simulink model "HDVSim_BEV_WPT_Truck"  (R2018b)
settings: Solver=ode3  StartTime=0.0  StopTime=inf  FixedStep=sample_time  MaxStep=0.1  RelTol=1e-3  EnableMultiTasking=on
model workspace (MAT data in the model file):
  Anschaffungskosten = [3707764736; 2; 1; 1; 1; 1]
  Bat : struct (value not decoded)
  DrvTrn = 12
  Eigenschaften = [3707764736; 2; 1; 1; 2; 2]
  Electric_Truck = 1
  Elektro_LKW = 1
  Fahrzyklus = 2
  FuelCell : struct (value not decoded)
  Fueltype = 12
  Gewichte = [3707764736; 2; 1; 1; 3; 3]
  Hybrid_LKW = 1
  Hybrid_Truck = 1
  IZ12 = 3600
  Kraftstoff = 12
  SOC_T_EM_completely_available = 0.5
  SOC_addition = 0.4
  SOC_target = 0.85
  Sailing_without_drag_torque = 1
  MCOS : class17 (value not decoded)
  TCO_Anhaenger = 1
  TCO_Trailer = 1
  T_distance_LPS_down = 250
  T_distance_LPS_up = 250
  Temp1 = [1000 1100 1200 1300 1400 1500 1600 1700 1800 1900 2000 2100 ... (41 elements, 1x41)]
  VSim : struct (value not decoded)
  Vehicle = 'BEV_WPT'
  Vorausschauender_Tempomat = 1
  WPT : struct (value not decoded)
  acquisition_costs = [3707764736; 2; 1; 1; 3; 3]
  addition_for_critical_slope = 0.01
  ambient : struct (value not decoded)
  auxiliary_consumers : struct (value not decoded)
  critical_altitude_difference = 50
  cycle : struct (value not decoded)
  dcycle = 2
  distance_1_PPC = 175
  distance_2_PPC = 200
  distance_PPC_altitude_difference = 10000
  driver : struct (value not decoded)
  em : struct (value not decoded)
  em_M_max = 1563
  engine : struct (value not decoded)
  final_drive : struct (value not decoded)
  ifElectric = 1
  ifOptimized = 1
  look_ahead_PPC = 250
  max_distance = 358138
  name = 'Verbrauchssimulation_Elektro_WPT_LKW'
  propertie = [3707764736; 2; 1; 1; 4; 4]
  prospective_cruise_control = 1
  sample_time = 0.1
  tank : struct (value not decoded)
  tires : struct (value not decoded)
  transmission : struct (value not decoded)
  v_PPC_delta = 2.77777777778
  v_max_electrical_drive_only = 13.8888888889
  vehicle : struct (value not decoded)
  vehicle_F_brake_max = 309890.14
  MCOS : class17 (value not decoded)
  x = [1563 13 1 400 10.0500050682 4.34242437682 3 1 0 978 1339 2 ... (15 elements, 1x15)]
values from neighbouring files (symbols this model uses):
  transmission = Transmission_gearing(15.86, 7, 0, 0, final_drive.ratio)    [Parameterizing.m]
symbols that are objects in the model workspace (value not decoded): ambient, auxiliary_consumers, Bat, cycle, driver, em, final_drive, tires, vehicle, WPT
undefined symbols (not defined in the file or in its folder): Ion, Lithium
libraries: simulink x22, powerlib x4, electricdrivelib x1
(part 2 of 3)

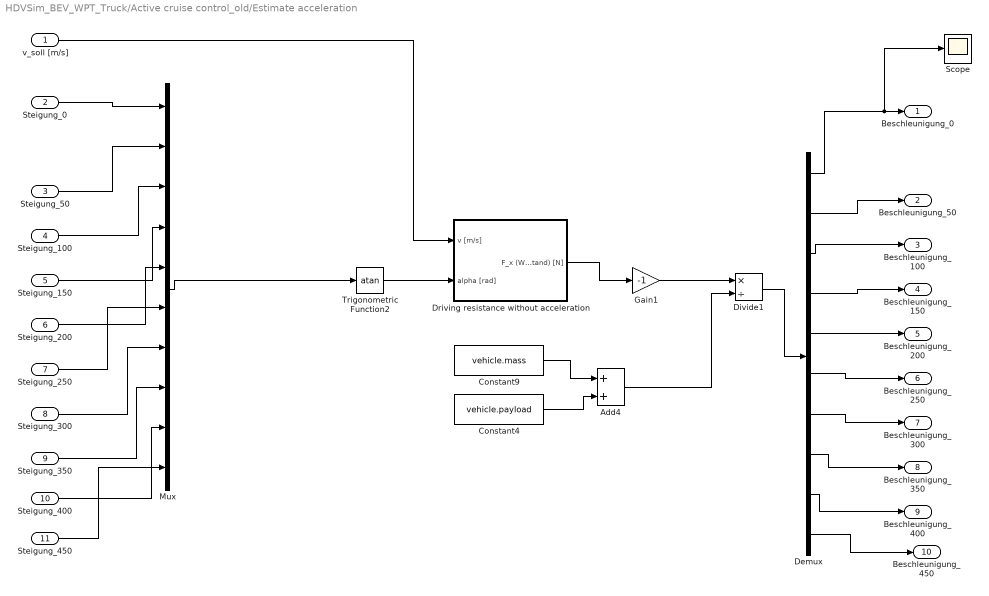
== system HDVSim_BEV_WPT_Truck/Active cruise control_old/Estimate acceleration ==
  v_soll [m/s] : Inport
  Steigung_0 : Inport  Port=2
  Steigung_50 : Inport  Port=3
  Steigung_100 : Inport  Port=4
  Steigung_150 : Inport  Port=5
  Steigung_200 : Inport  Port=6
  "Steigung_250 " : Inport  Port=7
  Steigung_300 : Inport  Port=8
  Steigung_350 : Inport  Port=9
  Steigung_400 : Inport  Port=10
  Steigung_450 : Inport  Port=11
  Add4 : Sum
  Constant4 : Constant  Value=vehicle.payload
  Constant9 : Constant  Value=vehicle.mass
  Demux : Demux  Outputs=10
  Divide1 : Product  Inputs=*/
  Driving resistance without acceleration : Subsystem
  Gain1 : Gain  Gain=-1
  Mux : Mux  Inputs=10
  Scope : Scope
  Trigonometric Function2 : Trigonometry  Operator=atan
  Beschleunigung_0 : Outport
  Beschleunigung_50 : Outport  Port=2
  Beschleunigung_100 : Outport  Port=3
  Beschleunigung_150 : Outport  Port=4
  Beschleunigung_200 : Outport  Port=5
  Beschleunigung_250 : Outport  Port=6
  Beschleunigung_300 : Outport  Port=7
  Beschleunigung_350 : Outport  Port=8
  Beschleunigung_400 : Outport  Port=9
  Beschleunigung_450 : Outport  Port=10
  wires:
  v_soll [m/s]/1 -> Driving resistance without acceleration/1(v [m/s])
  Trigonometric Function2/1 -> Driving resistance without acceleration/2(alpha [rad])
  Constant9/1 -> Add4/1
  Constant4/1 -> Add4/2
  Driving resistance without acceleration/1(F_x (Widerstand) [N]) -> Gain1/1
  Add4/1 -> Divide1/2
  Gain1/1 -> Divide1/1
  Mux/1 -> Trigonometric Function2/1
  Divide1/1 -> Demux/1
  Demux/1 -> Scope/1, Beschleunigung_0/1
  Steigung_0/1 -> Mux/1
  Steigung_50/1 -> Mux/2
  Steigung_100/1 -> Mux/3
  Steigung_150/1 -> Mux/4
  Steigung_200/1 -> Mux/5
  "Steigung_250 "/1 -> Mux/6
  Steigung_300/1 -> Mux/7
  Steigung_350/1 -> Mux/8
  Steigung_400/1 -> Mux/9
  Steigung_450/1 -> Mux/10
  Demux/2 -> Beschleunigung_50/1
  Demux/3 -> Beschleunigung_100/1
  Demux/4 -> Beschleunigung_150/1
  Demux/5 -> Beschleunigung_200/1
  Demux/6 -> Beschleunigung_250/1
  Demux/7 -> Beschleunigung_300/1
  Demux/8 -> Beschleunigung_350/1
  Demux/9 -> Beschleunigung_400/1
  Demux/10 -> Beschleunigung_450/1

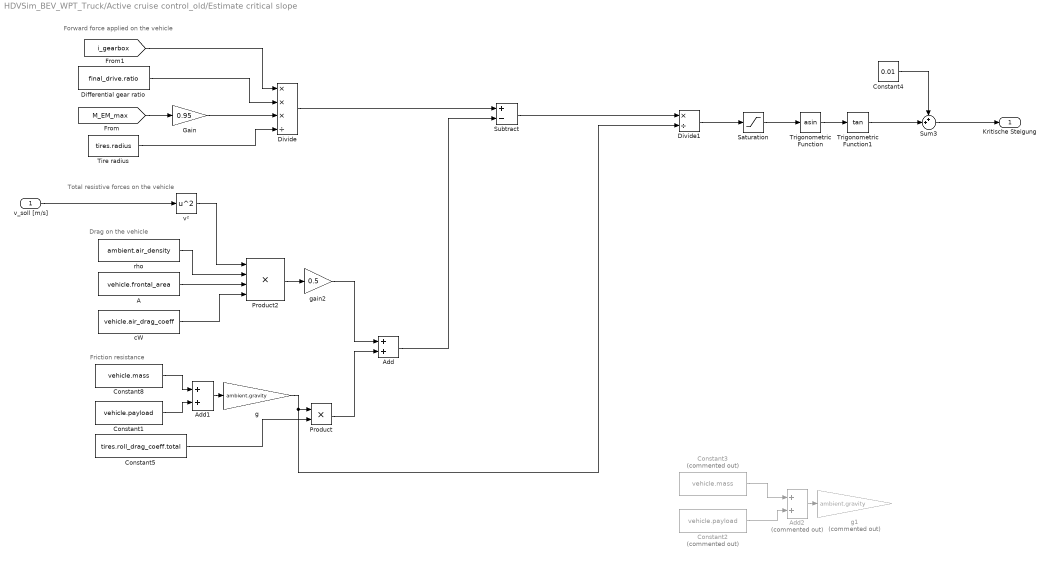
== system HDVSim_BEV_WPT_Truck/Active cruise control_old/Estimate critical slope ==
  note: Forward force applied on the vehicle
  note: Total resistive forces on the vehicle
  note: Drag on the vehicle
  note: Friction resistance
  v_soll [m/s] : Inport
  A : Constant  Value=vehicle.frontal_area
  Add : Sum
  Add1 : Sum
  Add2 : Sum  Commented=on
  Constant1 : Constant  Value=vehicle.payload
  Constant2 : Constant  Commented=on  Value=vehicle.payload
  Constant3 : Constant  Commented=on  Value=vehicle.mass
  Constant4 : Constant  Value=0.01
  Constant5 : Constant  Value=tires.roll_drag_coeff.total
  Constant8 : Constant  Value=vehicle.mass
  Differential gear ratio : Constant  Value=final_drive.ratio
  Divide : Product  Inputs=***/
  Divide1 : Product  Inputs=*/
  Gain : Gain  Gain=0.95
  Product : Product
  Product2 : Product  Inputs=4  RndMeth=Zero
  Saturation : Saturate  UpperLimit=1  LowerLimit=-1
  Subtract : Sum  Inputs=+-
  Sum3 : Sum  Inputs=++|
  Tire radius : Constant  Value=tires.radius
  Trigonometric Function : Trigonometry  Operator=asin
  Trigonometric Function1 : Trigonometry  Operator=tan
  cW : Constant  Value=vehicle.air_drag_coeff
  g : Gain  Gain=ambient.gravity
  g1 : Gain  Commented=on  Gain=ambient.gravity
  gain2 : Gain  Gain=0.5
  rho : Constant  Value=ambient.air_density
  v² : Math  Operator=square  SaturateOnIntegerOverflow=on
  Kritische Steigung : Outport
  wires:
  Gain/1 -> Divide/3
  [i_gearbox] -> Divide/1
  Differential gear ratio/1 -> Divide/2
  Tire radius/1 -> Divide/4
  Divide/1 -> Subtract/1
  v²/1 -> Product2/1
  rho/1 -> Product2/2
  A/1 -> Product2/3
  cW/1 -> Product2/4
  Product2/1 -> gain2/1
  Constant3/1 -> Add2/1
  Constant2/1 -> Add2/2
  Add2/1 -> g1/1
  Trigonometric Function/1 -> Trigonometric Function1/1
  Divide1/1 -> Saturation/1
  Sum3/1 -> Kritische Steigung/1
  [M_EM_max] -> Gain/1
  Constant4/1 -> Sum3/1
  Trigonometric Function1/1 -> Sum3/2
  v_soll [m/s]/1 -> v²/1
  Saturation/1 -> Trigonometric Function/1
  Add1/1 -> g/1
  Constant1/1 -> Add1/2
  g/1 -> Divide1/2, Product/1
  Constant5/1 -> Product/2
  Constant8/1 -> Add1/1
  Product/1 -> Add/2
  gain2/1 -> Add/1
  Add/1 -> Subtract/2
  Subtract/1 -> Divide1/1

== system HDVSim_BEV_WPT_Truck/Driver controls/PI controller brake1 ==
  delta_v : Inport
  Add : Sum
  "I - controller " : Gain  Gain=driver.controller_integral_gain
  "Integrator, reset for sign change of delta v" : Integrator  ExternalReset=either  ZeroCross=off
  "P - controller " : Gain  Gain=driver.controller_proportional_gain
  F_target [N] : Outport
  wires:
  "Integrator, reset for sign change of delta v"/1 -> Add/2  "I_part"
  "I - controller "/1 -> "Integrator, reset for sign change of delta v"/1
  Add/1 -> F_target [N]/1  "F_target"
  "P - controller "/1 -> Add/1  "P_part"
  delta_v/1 -> "I - controller "/1, "Integrator, reset for sign change of delta v"/2, "P - controller "/1

== system HDVSim_BEV_WPT_Truck/Driver controls/PI controller throttle1 ==
  delta_v : Inport
  Add : Sum
  "I - controller " : Gain  Gain=driver.controller_integral_gain
  "Integrator, reset for sign change of delta v" : Integrator  ExternalReset=either  ZeroCross=off
  "P - controller " : Gain  Gain=driver.controller_proportional_gain
  F_target [N] : Outport
  wires:
  "Integrator, reset for sign change of delta v"/1 -> Add/2  "I_part"
  "I - controller "/1 -> "Integrator, reset for sign change of delta v"/1
  Add/1 -> F_target [N]/1  "F_target"
  "P - controller "/1 -> Add/1  "P_partl"
  delta_v/1 -> "Integrator, reset for sign change of delta v"/2, "I - controller "/1, "P - controller "/1

== system HDVSim_BEV_WPT_Truck/Driver controls/ZKU ==
  In1 : Inport
  Switch1 : Switch  Threshold=1
  zero1 : Constant  Value=0
  Out1 : Outport
  wires:
  zero1/1 -> Switch1/1
  [ZKU] -> Switch1/2
  Switch1/1 -> Out1/1
  In1/1 -> Switch1/3

== system HDVSim_BEV_WPT_Truck/Driver controls/ZKU1 ==
  In1 : Inport
  Switch1 : Switch  Threshold=1
  zero1 : Constant  Value=0
  Out1 : Outport
  wires:
  In1/1 -> Switch1/3
  Switch1/1 -> Out1/1
  [ZKU] -> Switch1/2
  zero1/1 -> Switch1/1

== system HDVSim_BEV_WPT_Truck/Driving cycle/Holding time at the beginning ==
  In1 : Inport
  Clock2 : Clock
  Constant3 : Constant  Value=cycle.stop_start
  Constant4 : Constant  Value=0
  Subtract1 : Sum  Inputs=+-
  Switch1 : Switch
  Out1 : Outport
  wires:
  In1/1 -> Switch1/1
  Constant3/1 -> Subtract1/2
  Clock2/1 -> Subtract1/1
  Constant4/1 -> Switch1/3
  Subtract1/1 -> Switch1/2
  Switch1/1 -> Out1/1

== system HDVSim_BEV_WPT_Truck/Driving cycle/Holding time at the end ==
  In1 : Inport
  Clock1 : Clock
  Constant : Constant
  Constant1 : Constant  Value=cycle.stop_end
  Constant2 : Constant
  Hit Crossing : HitCross  HitCrossingDirection=rising
  Integrator : Integrator  ExternalReset=rising
  Subtract : Sum  Inputs=+-
  Sum1 : Sum  Inputs=|++
  Switch : Switch  Criteria=u2 > Threshold  Threshold=cycle.stop_end
  Out1 : Outport
  wires:
  In1/1 -> Integrator/2
  Constant/1 -> Integrator/1
  Subtract/1 -> Hit Crossing/1
  Integrator/1 -> Switch/1
  Hit Crossing/1 -> Out1/1
  Clock1/1 -> Switch/2
  Constant1/1 -> Sum1/2, Subtract/2
  Sum1/1 -> Switch/3
  Switch/1 -> Subtract/1
  Constant2/1 -> Sum1/1

== system HDVSim_BEV_WPT_Truck/Driving cycle/Significant speed deviation ==
  In1 : Inport
  Clock : Clock
  Compare To Constant : simulink/Logic and Bit Operations/Compare To Constant  relop=>=  const=30  OutDataTypeStr=boolean
  Compare To Constant1 : simulink/Logic and Bit Operations/Compare To Constant  relop=>=  const=1000  OutDataTypeStr=boolean
  Compare To Constant2 : simulink/Logic and Bit Operations/Compare To Constant  relop=<=  const=0.1  OutDataTypeStr=boolean
  Compare To Constant3 : simulink/Logic and Bit Operations/Compare To Constant  relop=>=  const=1000  OutDataTypeStr=boolean
  Constant : Constant
  Constant1 : Constant  Value=0
  Constant2 : Constant
  Constant3 : Constant  Value=0
  Display : Display
  Display1 : Display
  Display2 : Display
  Gain : Gain  Gain=3.6
  Gain1 : Gain  Gain=3.6
  Integrator1 : Integrator  ExternalReset=either
  Integrator2 : Integrator  ExternalReset=either
  Logical Operator : Logic  Operator=OR  AllPortsSameDT=off  OutDataTypeStr=boolean
  Sum : Sum  Inputs=|+-
  Switch : Switch  Criteria=u2 > Threshold
  Switch1 : Switch  Criteria=u2 > Threshold
  stop_time : Lookup_n-D  NumberOfTableDimensions=1  BreakpointsForDimension1=cycle.distance  Table=cycle.stop_time  InterpMethod=Flat  RndMeth=Simplest  InputSameDT=on
  Out1 : Outport
  wires:
  In1/1 -> stop_time/1
  stop_time/1 -> Sum/2
  [delta_v] -> Gain/1
  [v_current1] -> Gain1/1
  Gain/1 -> Compare To Constant/1
  Integrator2/1 -> Display/1, Compare To Constant1/1
  Compare To Constant1/1 -> Logical Operator/2
  Gain1/1 -> Compare To Constant2/1
  Integrator1/1 -> Sum/1
  Sum/1 -> Display1/1, Compare To Constant3/1
  Compare To Constant3/1 -> Logical Operator/1
  Logical Operator/1 -> Out1/1
  Switch1/1 -> Integrator2/1, Integrator2/2
  Switch/1 -> Integrator1/2, Integrator1/1
  Constant/1 -> Switch/1
  Compare To Constant2/1 -> Switch/2
  Constant1/1 -> Switch/3
  Constant2/1 -> Switch1/1
  Compare To Constant/1 -> Switch1/2
  Constant3/1 -> Switch1/3
  Clock/1 -> Display2/1

== system HDVSim_BEV_WPT_Truck/ECU/Approach to stop ==
  stop_time [s] : Inport
  Clock : Clock
  Compare To Constant1 : simulink/Logic and Bit Operations/Compare To Constant  relop=<=  const=3  OutDataTypeStr=boolean
  Compare To Zero : simulink/Logic and Bit Operations/Compare To Zero  relop=<=  OutDataTypeStr=boolean
  Constant : Constant
  Constant1 : Constant  Value=3.1
  Constant2 : Constant
  Hit Crossing : HitCross  HitCrossingDirection=rising
  Integrator : Integrator  ExternalReset=rising  InitialCondition=0.001
  Integrator1 : Integrator  ExternalReset=rising
  Scope : Scope  NumInputPorts=2
  Subtract : Sum  Inputs=+-
  Switch : Switch  Criteria=u2 > Threshold  Threshold=3
  Driver_M : Outport
  Driver_B : Outport  Port=2
  wires:
  Constant/1 -> Integrator/1
  stop_time [s]/1 -> Integrator/2, Subtract/2
  Integrator/1 -> Subtract/1
  Subtract/1 -> Compare To Zero/1, Hit Crossing/1
  Constant2/1 -> Integrator1/1
  Hit Crossing/1 -> Integrator1/2
  Clock/1 -> Switch/2
  Constant1/1 -> Switch/3
  Integrator1/1 -> Switch/1
  Switch/1 -> Compare To Constant1/1
  Compare To Constant1/1 -> Scope/1, Driver_M/1
  Compare To Zero/1 -> Scope/2, Driver_B/1

== system HDVSim_BEV_WPT_Truck/ECU/Calculations for braking ==
  M_EM_max [Nm] : Inport
  Driver_B : Inport  Port=2
  Gear : Inport  Port=3
  Differential efficiency : Constant  Value=final_drive.trq_eff
  Differential gear ratio : Constant  Value=final_drive.ratio
  Divide : Product  Inputs=*/
  Divide1 : Product  Inputs=**/*/
  Efficiencies : Lookup  InputValues=[1:length(transmission.ratios)]  Table=transmission.trq_eff  LookUpMeth=Use Input Nearest
  Gearing : Lookup  InputValues=[1:length(transmission.ratios)]  Table=transmission.ratios  LookUpMeth=Use Input Nearest
  Maximum breaking force [N] : Constant  Value=vehicle.F_brake_max
  Product : Product
  Time radius : Constant  Value=tires.radius
  F_brake_required [N] : Outport
  F_brake_elec_max [N] : Outport  Port=2
  wires:
  M_EM_max [Nm]/1 -> Divide1/1
  Divide/1 -> F_brake_elec_max [N]/1
  Divide1/1 -> Divide/1
  Time radius/1 -> Divide/2
  Driver_B/1 -> Product/1
  Maximum breaking force [N]/1 -> Product/2
  Product/1 -> F_brake_required [N]/1
  Gear/1 -> Efficiencies/1, Gearing/1
  Gearing/1 -> Divide1/2
  Efficiencies/1 -> Divide1/3
  Differential gear ratio/1 -> Divide1/4
  Differential efficiency/1 -> Divide1/5

== system HDVSim_BEV_WPT_Truck/ECU/Calculations for throttle ==
  Driver_M : Inport
  SOC : Inport  Port=2
  n_EM [rpm] : Inport  Port=3
  Memory1 : Memory
  Product : Product
  Product1 : Product
  Speed-torque characteristics : Lookup_n-D  NumberOfTableDimensions=1  BreakpointsForDimension1=em.speed  Table=em.trq  ExtrapMethod=Clip  RndMeth=Simplest  InputSameDT=on
  M_EM_max [Nm] : Outport
  M_Required [Nm] : Outport  Port=2
  wires:
  Product1/1 -> Product/2
  Driver_M/1 -> Product/1
  Product/1 -> M_Required [Nm]/1
  Speed-torque characteristics/1 -> Product1/1, M_EM_max [Nm]/1
  SOC/1 -> Product1/2
  n_EM [rpm]/1 -> Memory1/1
  Memory1/1 -> Speed-torque characteristics/1

== system HDVSim_BEV_WPT_Truck/Electric motor/Machine current demand ==
  note: Electric power calculation
  "n_EM " : Inport
  M_EM_target [Nm] : Inport  Port=2
  Eff_EM : Inport  Port=3
  U_Bat [V] : Inport  Port=4
  Divide : Product  Inputs=*/*
  Divide1 : Product  Inputs=*/
  Gain : Gain  Gain=2*pi/60
  Saturation1 : Saturate  UpperLimit=inf  LowerLimit=1
  I_target [A] : Outport
  wires:
  Divide/1 -> Divide1/1
  Divide1/1 -> I_target [A]/1
  Gain/1 -> Divide/3
  M_EM_target [Nm]/1 -> Divide/1
  "n_EM "/1 -> Saturation1/1
  U_Bat [V]/1 -> Divide1/2
  Eff_EM/1 -> Divide/2
  Saturation1/1 -> Gain/1

== system HDVSim_BEV_WPT_Truck/Electric motor/Machine torque demand ==
  n_EM [rpm] : Inport
  I_current [A] : Inport  Port=2
  Eff_EM : Inport  Port=3
  U_Bat [V] : Inport  Port=4
  Divide : Product  Inputs=*/*
  Gain : Gain  Gain=2*pi/60
  Product : Product
  Saturation1 : Saturate  UpperLimit=inf  LowerLimit=1
  M_EM [Nm] : Outport
  wires:
  U_Bat [V]/1 -> Product/1
  n_EM [rpm]/1 -> Saturation1/1
  Product/1 -> Divide/1
  Gain/1 -> Divide/2
  Divide/1 -> M_EM [Nm]/1
  I_current [A]/1 -> Product/2
  Eff_EM/1 -> Divide/3
  Saturation1/1 -> Gain/1

== system HDVSim_BEV_WPT_Truck/Power electronics and battery/Conversion of SOC ==
  SOC_absolut : Inport
  Constant : Constant  Value=0.5-Bat.Useable_range/2
  Constant1 : Constant  Value=0.5+Bat.Useable_range/2
  Divide : Product  Inputs=*/
  Saturation : Saturate  UpperLimit=1  LowerLimit=0
  Sum : Sum  Inputs=+-
  Sum1 : Sum  Inputs=-+
  SOC_relative : Outport
  wires:
  SOC_absolut/1 -> Sum/1
  Constant/1 -> Sum1/1, Sum/2
  Sum/1 -> Divide/1
  Constant1/1 -> Sum1/2
  Sum1/1 -> Divide/2
  Divide/1 -> Saturation/1
  Saturation/1 -> SOC_relative/1

== system HDVSim_BEV_WPT_Truck/Power electronics and battery/Power electronics ==
  I_PE_current [A] : Inport
  1/Eta_LE : Gain  Gain=1/Bat.Efficiency_power_electronics
  Eta_LE : Gain  Gain=Bat.Efficiency_power_electronics
  Switch : Switch  Criteria=u2 > Threshold
  I_BAT_current [A] : Outport
  wires:
  Eta_LE/1 -> Switch/1
  1/Eta_LE/1 -> Switch/3
  I_PE_current [A]/1 -> 1/Eta_LE/1, Eta_LE/1, Switch/2
  Switch/1 -> I_BAT_current [A]/1

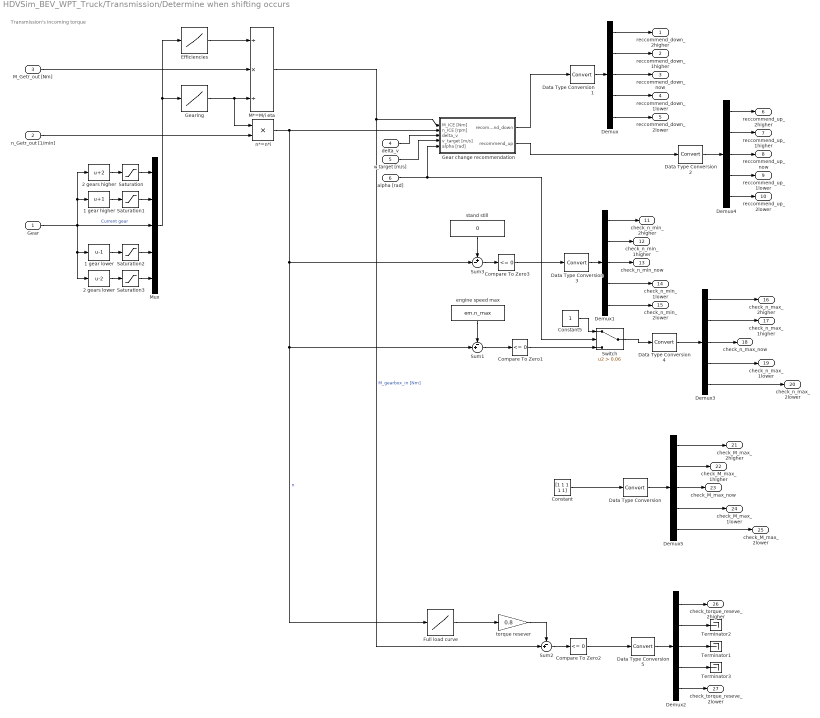
== system HDVSim_BEV_WPT_Truck/Transmission/Determine when shifting occurs ==
  note: Transmission's incoming torque
  Gear : Inport
  n_Getr_out [1/min] : Inport  Port=2
  M_Getr_out [Nm] : Inport  Port=3
  delta_v : Inport  Port=4
  v_target [m/s] : Inport  Port=5
  alpha [rad] : Inport  Port=6
  1 gear higher : Bias  Bias=1
  1 gear lower : Bias  Bias=-1
  2 gears higher : Bias  Bias=2
  2 gears lower : Bias  Bias=-2
  Compare To Zero1 : simulink/Logic and Bit Operations/Compare To Zero  relop=<=  OutDataTypeStr=boolean
  Compare To Zero2 : simulink/Logic and Bit Operations/Compare To Zero  relop=<=  OutDataTypeStr=boolean
  Compare To Zero3 : simulink/Logic and Bit Operations/Compare To Zero  relop=<=  OutDataTypeStr=boolean
  Constant : Constant  Value=[1 1 1 1 1]
  Constant5 : Constant
  Data Type Conversion{,1..5} (6 alike) : DataTypeConversion
  Demux{,1..5} (6 alike) : Demux  Outputs=5
  Efficiencies : Lookup  InputValues=[1:length(transmission.ratios)]  Table=transmission.trq_eff  LookUpMeth=Use Input Nearest
  Full load curve : Lookup  InputValues=em.speed  Table=em.trq  LookUpMeth=Interpolation-Use End Values
  Gear change recommendation : Subsystem
  Gearing : Lookup  InputValues=[1:length(transmission.ratios)]  Table=transmission.ratios  LookUpMeth=Use Input Nearest
  M*=M/i eta : Product  Inputs=/*/
  Mux : Mux  Inputs=5
  Saturation{,1..3} (4 alike) : Saturate  UpperLimit=length(transmission.ratios)  LowerLimit=0
  Sum1 : Sum  Inputs=-+|
  Sum2 : Sum  Inputs=-+|
  Sum3 : Sum  Inputs=+-|
  Switch : Switch  Criteria=u2 > Threshold  Threshold=0.06
  Terminator1 : Terminator
  Terminator2 : Terminator
  Terminator3 : Terminator
  engine speed max : Constant  Value=em.n_max
  n*=n*i : Product  Inputs=**
  stand still : Constant  Value=0
  torque resever : Gain  Gain=0.8
  reccommend_down_2higher : Outport
  reccommend_down_1higher : Outport  Port=2
  reccommend_down_now : Outport  Port=3
  reccommend_down_1lower : Outport  Port=4
  reccommend_down_2lower : Outport  Port=5
  reccommend_up_2higher : Outport  Port=6
  reccommend_up_1higher : Outport  Port=7
  reccommend_up_now : Outport  Port=8
  reccommend_up_1lower : Outport  Port=9
  reccommend_up_2lower : Outport  Port=10
  check_n_min_2higher : Outport  Port=11
  check_n_min_1higher : Outport  Port=12
  check_n_min_now : Outport  Port=13
  check_n_min_1lower : Outport  Port=14
  check_n_min_2lower : Outport  Port=15
  check_n_max_2higher : Outport  Port=16
  check_n_max_1higher : Outport  Port=17
  check_n_max_now : Outport  Port=18
  check_n_max_1lower : Outport  Port=19
  check_n_max_2lower : Outport  Port=20
  check_M_max_2higher : Outport  Port=21
  check_M_max_1higher : Outport  Port=22
  check_M_max_now : Outport  Port=23
  check_M_max_1lower : Outport  Port=24
  check_M_max_2lower : Outport  Port=25
  check_torque_reseve_2higher : Outport  Port=26
  check_torque_reseve_2lower : Outport  Port=27
  wires:
  Gear/1 -> 1 gear higher/1, 2 gears higher/1, Mux/3, 1 gear lower/1, 2 gears lower/1  "Current gear"
  n*=n*i/1 -> Full load curve/1, Sum1/2, Sum3/2, Gear change recommendation/2(n_ICE [rpm])  "n"
  n_Getr_out [1/min]/1 -> n*=n*i/2
  Efficiencies/1 -> M*=M/i eta/1
  M_Getr_out [Nm]/1 -> M*=M/i eta/2
  2 gears higher/1 -> Saturation/1
  1 gear higher/1 -> Saturation1/1
  Mux/1 -> Efficiencies/1, Gearing/1
  Demux/1 -> reccommend_down_2higher/1
  Demux/2 -> reccommend_down_1higher/1
  Demux/3 -> reccommend_down_now/1
  Demux2/1 -> check_torque_reseve_2higher/1
  Demux2/2 -> Terminator2/1
  Demux2/3 -> Terminator1/1
  Demux1/1 -> check_n_min_2higher/1
  Demux1/2 -> check_n_min_1higher/1
  Demux1/3 -> check_n_min_now/1
  Gearing/1 -> n*=n*i/1, M*=M/i eta/3
  Saturation/1 -> Mux/1
  Saturation1/1 -> Mux/2
  1 gear lower/1 -> Saturation2/1
  Saturation2/1 -> Mux/4
  2 gears lower/1 -> Saturation3/1
  Saturation3/1 -> Mux/5
  Demux2/4 -> Terminator3/1
  Demux2/5 -> check_torque_reseve_2lower/1
  Demux/5 -> reccommend_down_2lower/1
  Demux/4 -> reccommend_down_1lower/1
  Demux1/4 -> check_n_min_1lower/1
  Demux1/5 -> check_n_min_2lower/1
  Demux4/1 -> reccommend_up_2higher/1
  Demux4/2 -> reccommend_up_1higher/1
  Demux4/3 -> reccommend_up_now/1
  Demux4/5 -> reccommend_up_2lower/1
  Demux4/4 -> reccommend_up_1lower/1
  Gear change recommendation/1(recommend_down) -> Data Type Conversion1/1
  Full load curve/1 -> torque resever/1
  torque resever/1 -> Sum2/1
  Sum2/1 -> Compare To Zero2/1
  Compare To Zero2/1 -> Data Type Conversion5/1
  Sum3/1 -> Compare To Zero3/1
  stand still/1 -> Sum3/1
  Compare To Zero3/1 -> Data Type Conversion3/1
  Gear change recommendation/2(recommend_up) -> Data Type Conversion2/1
  Demux3/1 -> check_n_max_2higher/1
  Demux3/2 -> check_n_max_1higher/1
  Demux3/3 -> check_n_max_now/1
  Demux3/4 -> check_n_max_1lower/1
  Demux3/5 -> check_n_max_2lower/1
  Sum1/1 -> Compare To Zero1/1
  engine speed max/1 -> Sum1/1
  Demux5/1 -> check_M_max_2higher/1
  Demux5/2 -> check_M_max_1higher/1
  Demux5/3 -> check_M_max_now/1
  Demux5/4 -> check_M_max_1lower/1
  Demux5/5 -> check_M_max_2lower/1
  Data Type Conversion1/1 -> Demux/1
  Data Type Conversion2/1 -> Demux4/1
  Data Type Conversion3/1 -> Demux1/1
  Data Type Conversion4/1 -> Demux3/1
  Data Type Conversion/1 -> Demux5/1
  Data Type Conversion5/1 -> Demux2/1
  Constant/1 -> Data Type Conversion/1
  M*=M/i eta/1 -> Gear change recommendation/1(M_ICE [Nm]), Sum2/2  "M_gearbox_in [Nm]"
  delta_v/1 -> Gear change recommendation/3(delta_v)
  v_target [m/s]/1 -> Gear change recommendation/4(v_target [m/s])
  alpha [rad]/1 -> Gear change recommendation/5(alpha [rad]), Switch/2
  Constant5/1 -> Switch/1
  Switch/1 -> Data Type Conversion4/1
  Compare To Zero1/1 -> Switch/3

== system HDVSim_BEV_WPT_Truck/Transmission/Torque flow through the transmission ==
  note: Outgoing torque from the transmission
  note: Rotational speed before and after the transmission
  M_gearbox_in [Nm] : Inport
  n_gearbox_out [1/min] : Inport  Port=2
  Gear : Inport  Port=3
  Divide : Product  Inputs=/
  Efficiencies : Lookup  InputValues=[1:length(transmission.ratios)]  Table=transmission.trq_eff  LookUpMeth=Use Input Nearest
  Gearing : Lookup  InputValues=[1:length(transmission.ratios)]  Table=transmission.ratios  LookUpMeth=Use Input Nearest
  M_Mot >= 0? : Switch  ZeroCross=off
  Product : Product  Inputs=***  RndMeth=Zero
  Rotating mass : Lookup  InputValues=[1:length(transmission.ratios)]  Table=transmission.lamda  LookUpMeth=Use Input Nearest
  n*=n*i : Product  Inputs=**
  n_gearbox_in [1/min] : Outport
  M_gearbox_out [Nm] : Outport  Port=2
  i_gearbox : Outport  Port=3
  tag scope: Eta_Transmission=global, lamda=global
  wires:
  Gearing/1 -> n*=n*i/1, i_gearbox/1, Product/3  "i_Getriebe"
  n*=n*i/1 -> n_gearbox_in [1/min]/1
  Rotating mass/1 -> [lamda]  "lambda"
  n_gearbox_out [1/min]/1 -> n*=n*i/2
  Gear/1 -> Rotating mass/1, Gearing/1, Efficiencies/1
  M_gearbox_in [Nm]/1 -> M_Mot >= 0?/2, Product/2
  Product/1 -> M_gearbox_out [Nm]/1
  Efficiencies/1 -> Divide/1, M_Mot >= 0?/1  "Eta_Getriebe"
  Divide/1 -> M_Mot >= 0?/3
  M_Mot >= 0?/1 -> [Eta_Transmission], Product/1

== system HDVSim_BEV_WPT_Truck/Transmission/ZKU ==
  Gear : Inport
  Compare : simulink/Logic and Bit Operations/Compare To Zero  relop=>=  OutDataTypeStr=uint8  ZeroCross=off
  Constant : Constant  Value=-1
  Constant1 : Constant  Value=0
  Data Type Conversion : DataTypeConversion
  Derivative : Derivative
  Integrator : Integrator  ExternalReset=either  InitialCondition=transmission.shift_time
  Manual Switch : ManualSwitch
  tag scope: ZKU=global
  wires:
  Constant/1 -> Integrator/1
  Derivative/1 -> Integrator/2
  Integrator/1 -> Compare/1
  Compare/1 -> Data Type Conversion/1
  Data Type Conversion/1 -> [ZKU]
  Constant1/1 -> Manual Switch/2
  Gear/1 -> Manual Switch/1
  Manual Switch/1 -> Derivative/1

== system HDVSim_BEV_WPT_Truck/Vehicle/Driving resistances without acceleration ==
  alpha [rad] : Inport
  v [m/s] : Inport  Port=2
  Add : Sum  Inputs=+++
  Drag : Subsystem
  Gradient resistance : Subsystem
  Rolling resistance : Subsystem
  F_W [N] : Outport
  tag scope: Air_drag=global, Rolling_drag=global
  wires:
  Drag/1(F_drag [N]) -> [Air_drag], Add/2
  Rolling resistance/1(F_Roll [N]) -> Add/1, [Rolling_drag]
  Add/1 -> F_W [N]/1
  v [m/s]/1 -> Drag/1(v [m/s])
  Gradient resistance/1(F_slope [N]) -> Add/3
  alpha [rad]/1 -> Gradient resistance/1(alpha [rad]), Rolling resistance/1(alpha [rad])

== system HDVSim_BEV_WPT_Truck/Active cruise control/Estimate acceleration/Driving resistance without acceleration ==
  v [m/s] : Inport
  alpha [rad] : Inport  Port=2
  Add : Sum  Inputs=+++
  "Drag " : Subsystem
  Gradient resistance : Subsystem
  Rolling resistance : Subsystem
  F_x (resist) [N] : Outport
  wires:
  "Drag "/1(F_drag [N]) -> Add/2
  Rolling resistance/1(F_Roll [N]) -> Add/1
  Add/1 -> F_x (resist) [N]/1  "F_W"
  v [m/s]/1 -> "Drag "/1(v [m/s])
  Gradient resistance/1(F_slope [N]) -> Add/3
  alpha [rad]/1 -> Gradient resistance/1(alpha [rad]), Rolling resistance/1(alpha [rad])

== system HDVSim_BEV_WPT_Truck/Active cruise control_old/Estimate acceleration/Driving resistance without acceleration ==
  v [m/s] : Inport
  alpha [rad] : Inport  Port=2
  Add : Sum  Inputs=+++
  "Drag " : Subsystem
  Gradient resistance : Subsystem
  Rolling resistance : Subsystem
  F_x (Widerstand) [N] : Outport
  wires:
  "Drag "/1(F_Luft [N]) -> Add/2
  Rolling resistance/1(F_Roll [N]) -> Add/1
  Add/1 -> F_x (Widerstand) [N]/1  "F_W"
  v [m/s]/1 -> "Drag "/1(v [m/s])
  Gradient resistance/1(F_Steig [N]) -> Add/3
  alpha [rad]/1 -> Gradient resistance/1(alpha [rad]), Rolling resistance/1(alpha [rad])

== system HDVSim_BEV_WPT_Truck/Transmission/Determine when shifting occurs/Gear change recommendation ==
  note: Gear change commands
  M_ICE [Nm] : Inport
  n_ICE [rpm] : Inport  Port=2
  delta_v : Inport  Port=3
  v_target [m/s] : Inport  Port=4
  alpha [rad] : Inport  Port=5
  Constant1 : Constant  Value=0
  Constant3 : Constant  Value=em.shift_parameter.n2
  Constant5 : Constant  Value=em.shift_parameter.n6
  M_max [Nm] : Constant  Value=em.shift_parameter.M_max
  Switch : Switch  Criteria=u2 > Threshold  Threshold=0.06
  recommend_down_calcbsfc1 : Subsystem
  recommend_up_calc_bsfc1 : Subsystem
  recommend_down : Outport
  recommend_up : Outport  Port=2
  wires:
  M_max [Nm]/1 -> recommend_up_calc_bsfc1/5(M_max [Nm])  "M_max"
  n_ICE [rpm]/1 -> recommend_up_calc_bsfc1/4(n), recommend_down_calcbsfc1/1(n)
  M_ICE [Nm]/1 -> recommend_up_calc_bsfc1/1(M)
  recommend_down_calcbsfc1/1(Out1) -> recommend_down/1
  Constant3/1 -> recommend_up_calc_bsfc1/3(n_2), recommend_down_calcbsfc1/2(n_2)
  Constant5/1 -> recommend_up_calc_bsfc1/2(n_6)
  delta_v/1 -> recommend_down_calcbsfc1/3(delta_v)
  v_target [m/s]/1 -> recommend_up_calc_bsfc1/6(v_target [m/s])
  Constant1/1 -> Switch/1
  recommend_up_calc_bsfc1/1(recommend_up) -> Switch/3
  Switch/1 -> recommend_up/1
  alpha [rad]/1 -> Switch/2

== system HDVSim_BEV_WPT_Truck/Vehicle/Driving resistances without acceleration/Drag ==
  note: F_Drag = 0.5 * V^2 * rho * A * c_d
  v [m/s] : Inport
  A : Constant  Value=vehicle.frontal_area
  Product2 : Product  Inputs=4  RndMeth=Zero
  c_d : Constant  Value=vehicle.air_drag_coeff
  gain2 : Gain  Gain=0.5
  rho : Constant  Value=ambient.air_density
  v² : Math  Operator=square  SaturateOnIntegerOverflow=on
  F_drag [N] : Outport
  wires:
  v²/1 -> Product2/1
  gain2/1 -> F_drag [N]/1
  v [m/s]/1 -> v²/1
  rho/1 -> Product2/2
  A/1 -> Product2/3
  c_d/1 -> Product2/4
  Product2/1 -> gain2/1

== system HDVSim_BEV_WPT_Truck/Vehicle/Driving resistances without acceleration/Gradient resistance ==
  note: F_slope = (m_tot * g) * sin(alpha)
  alpha [rad] : Inport
  Add : Sum
  Cargo weight : Constant  Value=vehicle.payload
  Product7 : Product  RndMeth=Zero
  Trigonometric Function : Trigonometry
  Vehicle weight : Constant  Value=vehicle.mass
  g : Gain  Gain=ambient.gravity
  F_slope [N] : Outport
  wires:
  alpha [rad]/1 -> Trigonometric Function/1
  Trigonometric Function/1 -> Product7/2
  Product7/1 -> F_slope [N]/1
  g/1 -> Product7/1
  Vehicle weight/1 -> Add/1
  Cargo weight/1 -> Add/2
  Add/1 -> g/1

== system HDVSim_BEV_WPT_Truck/Vehicle/Driving resistances without acceleration/Rolling resistance ==
  note: F_Roll = (m_tot * g) * mu * cos(alpha)
  alpha [rad] : Inport
  Add1 : Sum
  Cargo weight : Constant  Value=vehicle.payload
  Product7 : Product  Inputs=3  RndMeth=Zero
  Rolling friction coefficient : Constant  Value=tires.roll_drag_coeff.total
  Trigonometric Function : Trigonometry  Operator=cos
  Vehicle weight : Constant  Value=vehicle.mass
  g : Gain  Gain=ambient.gravity
  F_Roll [N] : Outport
  wires:
  alpha [rad]/1 -> Trigonometric Function/1
  Product7/1 -> F_Roll [N]/1
  Rolling friction coefficient/1 -> Product7/3
  Trigonometric Function/1 -> Product7/1
  g/1 -> Product7/2
  Vehicle weight/1 -> Add1/1
  Cargo weight/1 -> Add1/2
  Add1/1 -> g/1

== system HDVSim_BEV_WPT_Truck/Active cruise control/Estimate acceleration/Driving resistance without acceleration/Drag  ==
  note: F_Drag = 0.5 * V^2 * rho * A * c_d
  v [m/s] : Inport
  A : Constant  Value=vehicle.frontal_area
  Product2 : Product  Inputs=4  RndMeth=Zero
  c_d : Constant  Value=vehicle.air_drag_coeff(end)
  gain2 : Gain  Gain=0.5
  rho : Constant  Value=ambient.air_density
  v² : Math  Operator=square  SaturateOnIntegerOverflow=on
  F_drag [N] : Outport
  wires:
  v²/1 -> Product2/1
  gain2/1 -> F_drag [N]/1
  v [m/s]/1 -> v²/1
  rho/1 -> Product2/2
  A/1 -> Product2/3
  Product2/1 -> gain2/1
  c_d/1 -> Product2/4

== system HDVSim_BEV_WPT_Truck/Active cruise control/Estimate acceleration/Driving resistance without acceleration/Gradient resistance ==
  note: F_slope = (m_tot * g) * sin(alpha)
  alpha [rad] : Inport
  Add : Sum
  Constant1 : Constant  Value=vehicle.payload
  Constant2 : Constant  Value=vehicle.mass
  Product7 : Product  RndMeth=Zero
  Trigonometric Function : Trigonometry
  g : Gain  Gain=ambient.gravity
  F_slope [N] : Outport
  wires:
  alpha [rad]/1 -> Trigonometric Function/1
  Trigonometric Function/1 -> Product7/2
  Product7/1 -> F_slope [N]/1
  g/1 -> Product7/1
  Constant2/1 -> Add/1
  Constant1/1 -> Add/2
  Add/1 -> g/1

== system HDVSim_BEV_WPT_Truck/Active cruise control/Estimate acceleration/Driving resistance without acceleration/Rolling resistance ==
  note: F_Roll = (m_tot * g) * mu * cos(alpha)
  alpha [rad] : Inport
  Add1 : Sum
  Constant1 : Constant  Value=vehicle.payload
  Constant5 : Constant  Value=tires.roll_drag_coeff.total
  Constant8 : Constant  Value=vehicle.mass
  Product7 : Product  Inputs=3  RndMeth=Zero
  Trigonometric Function : Trigonometry  Operator=cos
  g : Gain  Gain=ambient.gravity
  F_Roll [N] : Outport
  wires:
  alpha [rad]/1 -> Trigonometric Function/1
  Product7/1 -> F_Roll [N]/1
  Constant5/1 -> Product7/3
  Trigonometric Function/1 -> Product7/1
  g/1 -> Product7/2
  Constant8/1 -> Add1/1
  Constant1/1 -> Add1/2
  Add1/1 -> g/1

== system HDVSim_BEV_WPT_Truck/Active cruise control_old/Estimate acceleration/Driving resistance without acceleration/Drag  ==
  note: F_Drag = 0.5 * V^2 * rho * A * c_d
  v [m/s] : Inport
  A : Constant  Value=vehicle.frontal_area
  Product2 : Product  Inputs=4  RndMeth=Zero
  cW : Constant  Value=vehicle.air_drag_coeff
  gain2 : Gain  Gain=0.5
  rho : Constant  Value=ambient.air_density
  v² : Math  Operator=square  SaturateOnIntegerOverflow=on
  F_Luft [N] : Outport
  wires:
  v²/1 -> Product2/1
  gain2/1 -> F_Luft [N]/1
  v [m/s]/1 -> v²/1
  rho/1 -> Product2/2
  A/1 -> Product2/3
  cW/1 -> Product2/4
  Product2/1 -> gain2/1

== system HDVSim_BEV_WPT_Truck/Active cruise control_old/Estimate acceleration/Driving resistance without acceleration/Gradient resistance ==
  note: F_slope = (m_tot * g) * sin(alpha)
  alpha [rad] : Inport
  Add : Sum
  Constant1 : Constant  Value=vehicle.payload
  Constant2 : Constant  Value=vehicle.mass
  Product7 : Product  RndMeth=Zero
  Trigonometric Function : Trigonometry
  g : Gain  Gain=ambient.gravity
  F_Steig [N] : Outport
  wires:
  alpha [rad]/1 -> Trigonometric Function/1
  Trigonometric Function/1 -> Product7/2
  Product7/1 -> F_Steig [N]/1
  g/1 -> Product7/1
  Constant2/1 -> Add/1
  Constant1/1 -> Add/2
  Add/1 -> g/1

== system HDVSim_BEV_WPT_Truck/Active cruise control_old/Estimate acceleration/Driving resistance without acceleration/Rolling resistance ==
  note: F_Roll = (m_tot * g) * mu * cos(alpha)
  alpha [rad] : Inport
  Add1 : Sum
  Constant1 : Constant  Value=vehicle.payload
  Constant5 : Constant  Value=tires.roll_drag_coeff.total
  Constant8 : Constant  Value=vehicle.mass
  Product7 : Product  Inputs=3  RndMeth=Zero
  Trigonometric Function : Trigonometry  Operator=cos
  g : Gain  Gain=ambient.gravity
  F_Roll [N] : Outport
  wires:
  alpha [rad]/1 -> Trigonometric Function/1
  Product7/1 -> F_Roll [N]/1
  Constant5/1 -> Product7/3
  Trigonometric Function/1 -> Product7/1
  g/1 -> Product7/2
  Constant8/1 -> Add1/1
  Constant1/1 -> Add1/2
  Add1/1 -> g/1

== system HDVSim_BEV_WPT_Truck/Transmission/Determine when shifting occurs/Gear change recommendation/recommend_down_calcbsfc1 ==
  n : Inport
  n_2 : Inport  Port=2
  delta_v : Inport  Port=3
  Compare To Constant : simulink/Logic and Bit Operations/Compare To Constant  relop=>=  const=8  OutDataTypeStr=boolean
  Compare To Constant1 : simulink/Logic and Bit Operations/Compare To Constant  relop=>=  const=5  OutDataTypeStr=boolean
  Compare To Zero3 : simulink/Logic and Bit Operations/Compare To Zero  relop=<=  OutDataTypeStr=boolean
  Constant : Constant  Value=em.n_eck
  Constant2 : Constant
  Constant3 : Constant  Value=0
  Display : Display
  Gain : Gain  Gain=3.6
  Gain1 : Gain  Gain=1.05
  Integrator2 : Integrator  ExternalReset=either
  Logical Operator : Logic  Operator=OR  AllPortsSameDT=off  OutDataTypeStr=boolean
  Logical Operator1 : Logic  AllPortsSameDT=off  OutDataTypeStr=boolean
  Relational Operator : RelationalOperator  Operator=<=  OutDataTypeStr=boolean  RndMeth=Nearest
  Scope : Scope  NumInputPorts=3
  Speed jump when shifting down (gear dependent) : Gain  Gain=transmission.ratios(1)/transmission.ratios(2)
  Sum1 : Sum  Inputs=+-|
  Switch1 : Switch  Criteria=u2 > Threshold
  Out1 : Outport
  wires:
  Sum1/1 -> Compare To Zero3/1
  n/1 -> Speed jump when shifting down (gear dependent)/1, Sum1/1
  n_2/1 -> Sum1/2
  Logical Operator/1 -> Scope/3, Out1/1
  Compare To Zero3/1 -> Logical Operator/1
  delta_v/1 -> Gain/1
  Gain/1 -> Compare To Constant/1
  Integrator2/1 -> Display/1, Compare To Constant1/1
  Switch1/1 -> Integrator2/1, Integrator2/2
  Constant2/1 -> Switch1/1
  Compare To Constant/1 -> Switch1/2
  Constant3/1 -> Switch1/3
  Compare To Constant1/1 -> Scope/2, Logical Operator1/1  "velocity too low"
  Logical Operator1/1 -> Logical Operator/2
  Speed jump when shifting down (gear dependent)/1 -> Relational Operator/1
  Constant/1 -> Gain1/1
  Gain1/1 -> Relational Operator/2
  Relational Operator/1 -> Scope/1, Logical Operator1/2  "Speed jump when shifting"

== system HDVSim_BEV_WPT_Truck/Transmission/Determine when shifting occurs/Gear change recommendation/recommend_up_calc_bsfc1 ==
  M : Inport
  n_6 : Inport  Port=2
  n_2 : Inport  Port=3
  n : Inport  Port=4
  M_max [Nm] : Inport  Port=5
  v_target [m/s] : Inport  Port=6
  Compare To Zero : simulink/Logic and Bit Operations/Compare To Zero  relop=<=  OutDataTypeStr=boolean
  Compare To Zero1 : simulink/Logic and Bit Operations/Compare To Zero  relop=~=  OutDataTypeStr=boolean
  Logical Operator : Logic  AllPortsSameDT=off  OutDataTypeStr=boolean
  MATLAB Function1 : MATLAB Function  TreatAsAtomicUnit=on
  Sum2 : Sum  Inputs=+-|
  recommend_up : Outport
  wires:
  n_6/1 -> MATLAB Function1/3(n_6)
  n/1 -> MATLAB Function1/1(n)
  n_2/1 -> MATLAB Function1/4(n_2)
  M_max [Nm]/1 -> MATLAB Function1/2(M_max)
  M/1 -> Sum2/1
  Sum2/1 -> Compare To Zero/1
  MATLAB Function1/1(g_2) -> Sum2/2
  v_target [m/s]/1 -> Compare To Zero1/1
  Compare To Zero/1 -> Logical Operator/1
  Compare To Zero1/1 -> Logical Operator/2
  Logical Operator/1 -> recommend_up/1

== stateflow chart HDVSim_BEV_WPT_Truck/ECU/Chart ==
  state Hybrid_Truck
    state Accelerating
      state Electric_driving
        during: u_EM = M_required / M_EM_max; u_KB = 0;
      (default) -> (junction 496)
      (junction 496) -> Electric_driving
    state Braking
      state Braking1
        % Conventional braking
        during: u_KB = Driver_B; u_EM = 0;
      state Braking2
        % Regenerative braking, additionally possibly conventional braking
        entry: REKUP = 1;
        exit: REKUP = 0;
        state Braking3
          % Regenerative and conventional braking
          during: u_EM = -1; u_KB = (F_brake_required - F_brake_el_max) / vehicle_F_brake_max;
        state Braking4
          % Regenerative braking
          during: u_EM = -(F_brake_required / F_brake_el_max); u_KB = 0;
        (junction 504) -> Braking3 [order 1] : [F_brake_required > F_brake_el_max]
        Braking4 -> Braking3 : [F_brake_required > F_brake_el_max]
        Braking3 -> Braking4 : [F_brake_required <= F_brake_el_max]
        (default) -> (junction 504)
        (junction 504) -> Braking4 [order 2] : [F_brake_required <= F_brake_el_max]
      (junction 497) -> Braking1 [order 1] : [SOC == 1]
      Braking2 -> Braking1 : [SOC == 1]
      Braking1 -> Braking2 : [SOC < 1]
      (default) -> (junction 497)
      (junction 497) -> Braking2 [order 2]
    state Rolling
      entry: ROLL = 1;
      exit: ROLL = 0;
      state Rolling1
        during: u_EM = 0; u_KB = 0;
      (default) -> Rolling1
    (junction 513) -> Accelerating [order 1] : [Driver_M > 0 && Driver_B == 0]
    (default) -> (junction 513)
    (junction 513) -> Braking [order 2] : [Driver_B > 0 && Driver_M == 0]
    (junction 513) -> Rolling [order 3]
    Braking -> Accelerating [order 1] : [Driver_M > 0 && Driver_B == 0]
    Accelerating -> Braking [order 1] : [Driver_B > 0 && Driver_M == 0]
    Rolling -> Accelerating [order 2] : [Driver_M > 0 && Driver_B == 0]
    Accelerating -> Rolling [order 2] : [Driver_M == 0 && Driver_B == 0]
    Rolling -> Braking [order 1] : [Driver_B > 0 && Driver_M == 0]
    Braking -> Rolling [order 2] : [Driver_M == 0 && Driver_B == 0]
  (junction 222) -> Hybrid_Truck : [Hybrid_Truck == 1]
  (default) -> (junction 222)
  data: u_EM output; Driver_M input; Driver_B input; SOC input; M_EM_max input; M_required input; F_brake_required input; F_brake_el_max input; u_KB output; Hybrid_Truck parameter; ROLL output; REKUP output; vehicle_F_brake_max local =vehicle.F_brake_max; em_M_max local double =em.M_max

== stateflow chart HDVSim_BEV_WPT_Truck/Active cruise control_old/Chart ==
  state Steigung
    entry: Signal_Steigung = 1;
    during: v_vT = 6/3.6;
    exit: Signal_Steigung = 0;
  state Ebene
    during: Signal_Steigung = 0; v_vT = 0;
  state Gefaelle1
    entry: Signal_Gefaelle = 1;
    exit: Signal_Gefaelle = 0;
  Ebene -> Steigung [order 2] : [Steigung_500 > Kritische_Steigung &&...
      | Steigung_700 > Kritische_Steigung &&...
      | Vorausschauender_Tempomat == 1]
  Steigung -> Ebene : [Steigung_50 <= Kritische_Steigung &&...
      | Steigung_0 <= Kritische_Steigung &&...
      | Zurueckgelegte_Strecke > 500]
  (default) -> Ebene
  Ebene -> Gefaelle1 [order 1] : [(Beschleunigung_0 +...
      | Beschleunigung_50 +...
      | Beschleunigung_100 +...
      | Beschleunigung_150 +...
      | Beschleunigung_200)/5 >= -0.17 &&...
      | (Beschleunigung_250 +...
      | Beschleunigung_300 +...
      | Beschleunigung_350 +...
      | Beschleunigung_400 +...
      | Beschleunigung_450)/5 >= 0.17 &&...
      | Fahrer_M > 0 &&...
      | Vorausschauender_Tempomat == 1]
  Gefaelle1 -> Ebene : [Zurueckgelegte_Strecke_2 > 450 ||...
      | Fahrer_B > 0]
  data: Kritische_Steigung input; Beschleunigung_0 input; Beschleunigung_50 input; Beschleunigung_100 input; Beschleunigung_150 input; Beschleunigung_200 input; Beschleunigung_250 input; Beschleunigung_300 input; Beschleunigung_350 input; Beschleunigung_400 input; Beschleunigung_450 input; Steigung_0 input; Steigung_50 input; Steigung_100 input; Steigung_250 input; Steigung_350 input; Steigung_500 input; Steigung_700 input; Fahrer_M input; Fahrer_B input; Zurueckgelegte_Strecke input; Zurueckgelegte_Strecke_2 input; v_vT output double; Signal_Steigung output double; Signal_Gefaelle output double; Vorausschauender_Tempomat parameter

== stateflow chart HDVSim_BEV_WPT_Truck/Transmission/Shifting rules ==
  state gear_state [parallel]
    settings: executionOrder=1
    state gear016
      entry: gear=16;
    state gear015
      entry: gear=15;
    state gear014
      entry: gear=14;
    state gear013
      entry: gear=13;
    state gear012
      entry: gear=12;
    state gear011
      entry: gear=11;
    state gear010
      entry: gear=10;
    state gear09
      entry: gear=9;
    state gear08
      entry: gear=8;
    state gear07
      entry: gear=7;
    state gear06
      entry: gear=6;
    state gear05
      entry: gear=5;
    state gear04
      entry: gear=4;
    state gear03
      entry: gear=3;
    state gear02
      entry: gear=2;
    state gear01
      entry: gear=1;
    gear015 -> gear016 [order 3] : TWO_UP
    gear016 -> gear014 [order 2] : TWO_DOWN
    gear014 -> gear016 [order 3] : TWO_UP
    (junction 349) -> gear016 [order 16] : [init_gear==16]
    gear016 -> gear015 [order 1] : DOWN
    gear015 -> gear016 [order 1] : UP
    gear015 -> gear013 [order 4] : TWO_DOWN
    (junction 349) -> gear015 [order 15] : [init_gear==15]
    gear013 -> gear015 [order 3] : TWO_UP
    gear015 -> gear014 [order 2] : DOWN
    gear014 -> gear015 [order 1] : UP
    gear014 -> gear012 [order 4] : TWO_DOWN
    gear012 -> gear014 [order 4] : TWO_UP
    (junction 349) -> gear014 [order 14] : [init_gear==14]
    gear014 -> gear013 [order 2] : DOWN
    gear013 -> gear014 [order 1] : UP
    gear011 -> gear013 [order 4] : TWO_UP
    gear013 -> gear011 [order 4] : TWO_DOWN
    (junction 349) -> gear013 [order 13] : [init_gear==13]
    gear013 -> gear012 [order 2] : DOWN
    gear012 -> gear013 [order 3] : UP
    (junction 349) -> gear012 [order 12] : [init_gear==12]
    gear010 -> gear012 [order 4] : TWO_UP
    gear012 -> gear010 [order 2] : TWO_DOWN
    gear012 -> gear011 [order 1] : DOWN
    gear011 -> gear012 [order 1] : UP
    gear011 -> gear09 [order 3] : TWO_DOWN
    gear09 -> gear011 [order 4] : TWO_UP
    (junction 349) -> gear011 [order 11] : [init_gear==11]
    gear011 -> gear010 [order 2] : DOWN
    gear010 -> gear011 [order 1] : UP
    gear08 -> gear010 [order 4] : TWO_UP
    gear010 -> gear08 [order 3] : TWO_DOWN
    (junction 349) -> gear010 [order 10] : [init_gear==10]
    gear010 -> gear09 [order 2] : DOWN
    gear09 -> gear010 [order 1] : UP
    gear07 -> gear09 [order 4] : TWO_UP
    gear09 -> gear07 [order 3] : TWO_DOWN
    (junction 349) -> gear09 [order 9] : [init_gear==9]
    gear09 -> gear08 [order 2] : DOWN
    gear08 -> gear09 [order 2] : UP
    gear06 -> gear08 [order 4] : TWO_UP
    gear08 -> gear06 [order 3] : TWO_DOWN
    (junction 349) -> gear08 [order 8] : [init_gear==8]
    gear08 -> gear07 [order 1] : DOWN
    gear07 -> gear08 [order 3] : UP
    gear07 -> gear05 [order 2] : TWO_DOWN
    gear05 -> gear07 [order 4] : TWO_UP
    (junction 349) -> gear07 [order 7] : [init_gear==7]
    gear07 -> gear06 [order 1] : DOWN
    gear06 -> gear07 [order 3] : UP
    (junction 349) -> gear06 [order 6] : [init_gear==6]
    gear06 -> gear04 [order 2] : TWO_DOWN
    (junction 349) -> gear01 [order 1] : [init_gear==1]
    (junction 349) -> gear05 [order 5] : [init_gear==5]
    gear04 -> gear06 [order 3] : TWO_UP
    (junction 349) -> gear02 [order 2] : [init_gear==2]
    (junction 349) -> gear04 [order 4] : [init_gear==4]
    (junction 349) -> gear03 [order 3] : [init_gear==3]
    gear06 -> gear05 [order 1] : DOWN
    gear05 -> gear06 [order 1] : UP
    gear05 -> gear03 [order 3] : TWO_DOWN
    gear03 -> gear05 [order 3] : TWO_UP
    gear05 -> gear04 [order 2] : DOWN
    gear04 -> gear05 [order 1] : UP
    gear04 -> gear02 [order 4] : TWO_DOWN
    gear02 -> gear04 [order 3] : TWO_UP
    gear04 -> gear03 [order 2] : DOWN
    gear03 -> gear04 [order 1] : UP
    gear01 -> gear03 [order 2] : TWO_UP
    gear03 -> gear01 [order 4] : TWO_DOWN
    gear03 -> gear02 [order 2] : DOWN
    gear02 -> gear03 [order 1] : UP
    gear02 -> gear01 [order 4] : TWO_DOWN
    gear02 -> gear01 [order 2] : DOWN
    gear01 -> gear02 [order 1] : UP
  state selection_state [parallel]
    settings: executionOrder=2
    state upshift2
      entry: TWO_UP;
      exit: wait_state.WAIT;
    state upshift1
      entry: UP;
      exit: wait_state.WAIT;
    note: 2: UP due to recommendation
        | 3: UP due to speed max
    state steady_state
    note: 1: DOWN due to recommendation
        | 4: DOWN due to torque maximum
        | 5: DOWN due to speed min
    state downshift1
      entry: DOWN;
      exit: wait_state.WAIT;
    state downshift2
      entry: TWO_DOWN;
      exit: wait_state.WAIT;
    upshift2 -> steady_state
    (junction 405) -> upshift2 [order 1] : [recommend_down_2higher==0]
    (junction 405) -> upshift1 [order 2] : [recommend_down_2higher==1]
    (junction 404) -> (junction 405) [order 1] : [check_torque_reserve_2higher==1]
    (junction 404) -> upshift1 [order 2] : [check_torque_reserve_2higher==0]
    (junction 403) -> (junction 404) [order 1] : [check_n_min_2higher==1 && check_M_max_2higher==1]
    (junction 403) -> upshift1 [order 2] : [check_n_min_2higher==0 || check_M_max_2higher==0]
    (junction 380) -> (junction 403) [order 1] : [recommend_up_1higher==1 && gear<=z-2]
    (junction 380) -> upshift1 [order 2] : [recommend_up_1higher==0 || gear>z-2]
    steady_state -> upshift1 [order 3] : [check_n_max_now==0 && gear<=z-1]
    upshift1 -> steady_state
    steady_state -> (junction 380) [order 2] : [(recommend_up_now==1 && check_n_max_now==1) && check_n_min_1higher==1 && check_M_max_1higher==1 && gear<=z-1 && waiting3s==0]
    (default) -> steady_state
    downshift1 -> steady_state
    downshift2 -> steady_state
    steady_state -> (junction 367) [order 1] : [(recommend_down_now==1 && check_M_max_now==1 && check_n_min_now==1) && check_n_max_1lower==1 && gear>=2 && waiting3s==0]
    steady_state -> downshift1 [order 4] : [(check_M_max_now==0 && check_n_min_now==1) && check_n_max_1lower==1 &&gear>=2 && check_n_min_now==1 && waiting3s==0]
    steady_state -> downshift1 [order 5] : [(check_n_min_now==0)&& gear>3]
    (junction 367) -> downshift1 [order 1] : [recommend_down_1lower==0 || gear<3]
    (junction 367) -> (junction 394) [order 2] : [recommend_down_1lower==1 && gear>=3]
    (junction 394) -> downshift1 [order 2] : [check_n_max_2lower==0]
    (junction 394) -> (junction 397) [order 1] : [check_n_max_2lower==1]
    (junction 397) -> downshift1 [order 2] : [recommend_up_2lower==1]
    (junction 397) -> downshift2 [order 1] : [recommend_up_2lower==0]
  state wait_state [parallel]
    settings: executionOrder=3
    state shifting_ready
      entry: waiting3s=0;
    state wait3s
      entry: waiting3s=1;
    shifting_ready -> wait3s : WAIT
    (default) -> shifting_ready
    wait3s -> shifting_ready : after(3,sec)
  (default) -> (junction 349)
  data: gear output uint8; waiting3s local double; init_gear input double; z input double; recommend_down_2higher input boolean; recommend_down_1higher input boolean; recommend_down_now input boolean; recommend_down_1lower input boolean; recommend_down_2llower input boolean; recommend_up_2higher input boolean; recommend_up_1higher input boolean; recommend_up_now input boolean; recommend_up_1lower input boolean; recommend_up_2lower input boolean; check_n_min_2higher input boolean; check_n_min_1higher input boolean; check_n_min_now input boolean; check_n_min_1lower input boolean; check_n_min_2lower input boolean; check_n_max_2higher input boolean; check_n_max_1higher input boolean; check_n_max_now input boolean; check_n_max_1lower input boolean; check_n_max_2lower input boolean; check_M_max_2higher input boolean; check_M_max_1higher input boolean; check_M_max_now input boolean; check_M_max_1lower input boolean; check_M_max_2lower input boolean; check_torque_reserve_2higher input boolean; check_torque_reserve_2lower input boolean
  events: WAIT local EITHER_EDGE_EVENT; DOWN local EITHER_EDGE_EVENT; TWO_DOWN local EITHER_EDGE_EVENT; TWO_UP local EITHER_EDGE_EVENT; UP local EITHER_EDGE_EVENT
